simulink model "TosAeb"  (R2021a)
settings: Solver=FixedStepDiscrete  StartTime=0.0  StopTime=inf  FixedStep=0.01  RelTol=1e-3
undefined symbols (not defined in the file or in its folder): ACCModSts, AccSts1, AEB_ObjectMovingOrientation, AEB_ObjectMovingState, CN_BTCActive, CN_CurrentGearR, CN_CurrentGearR_DHT, CN_PTCActive, CN_VDCActive, CN_VSC_EspOff, CN_VSC_FCW_AEB_Error, CN_VSC_FCW_AEB_NoDispay, CN_VSC_FCW_AEB_PerfmDgdtn, CN_VSC_FCW_AEB_TempUnAvlbl, CN_VSC_VehStandstill, CNaccelAvoidMax, CNaccelAvoidMin, CNbrakePedalPress, CNcomfortStrLeftAccelMax, CNcomfortStrLeftAccelMin, CNcomfortStrRightAccelMax, CNcomfortStrRightAccelMin, CNDrvDoorOpen, CNDrvModeEco, CNDrvModeNorm, CNDrvModeOther, CNDrvModeSport, CNDrvSBRreminder, CNNull, CNobjInvalidID, CNPassDoorOpen, CNPassSBRreminder, CNPEBSAccelNegInf, CNPEBSAccelNull, CNPEBSTimeInf, CNPEBSTimeNull, CNSTOPMAX, CNSTOPNULL, CNsubjWidth, CNSwtOFF, CNSwtON, CNTTCMAX, CNTTCNULL, CNVehConfigAEBCycNone, CNVehConfigAEBNone, CNVehConfigAEBPedNone, CNVehConfigFCWNone, CNVehConfigJANone, CollisionSeverity, FALSE, FCWSnvty, JACycObjCalcInfoDefaultParams, JAPedObjCalcInfoDefaultParams, JAVehObjCalcInfoDefaultParams, latCycObjCalcInfoDefaultParams, latPedObjCalcInfoDefaultParams, longObjCalcInfoDefaultParams, ModSts, movingStatus, ObjectType ... (+1112)
libraries: simulink x3031, AEBSFunctionLib x50, Ta_Library x33, AEBSStateMachineLib x23, Mylibrary x12, simulink_extras x7, ObjSelect_lib x3, dspstat3 x2
(part 24 of 102)

== system TosAeb/FcwAebJa_Step_sys/ALG_AEBSystem/ALG_AEBController/AEB_FuncCondCalcApp/LongFunctionCondCalc/vehAEBpartSuppAborCondCalc/vehAEBpartSuppCondCalc ==
  inputSignals : Inport
  Bus Selector : BusSelector  OutputSignals=longObjCalcInfo.LongObjRawInfo.ObjectMovingState,longObjCalcInfo.LongObjRawInfo.ObjectTypeCameraConfirmed,longObjCalcInfo.LongObjRawInfo.ObjectType,subjInfo.subjCurvTraj,subjInfo.subjLatAccel,subjInfo.subjVel,vehConfigInfo.VehicleConfigAEBCyc,vehConfigInfo.VehicleConfigAEBPed,vehStateCalcInfo.VSC_AEBFuncSwtSts.AEB_PedResp,longObjCalcInfo.LongObjRawInfo.ObjectMovingOrientation,longObjCalcInfo.LongObjRawInfo.ObjectID,longObjCalcInfo.LongObjRawInfo.ObjectExistProb,longObjCalcInfo.LongObjRawInfo.ObjectIsObstacleProb,longObjCalcInfo.LongObjRawInfo.dxVariance,longObjCalcInfo.longObjAbsInfo.dxFrontBumper,longObjCalcInfo.longObjAbsInfo.ObjFusQuality
  Bus Selector1 : BusSelector  OutputSignals=subjInfo.subjVel,vehCanInfo.SteerWheelAngle,vehCanInfo.SteerWheelSpd,vehCanInfo.AccrPedlPosnDiagc,vehConfigInfo.VehConfigIbooster,vehCanInfo.ESP_MasterCylindBrakePress,vehCanInfo.BrakePedalStatus_G,vehStateCalcInfo.VSCVehSuppAborCond.VSCvehAEBpartSuppCond,vehCanInfo.IB_BrkPedalAppld
  Bus Selector2 : BusSelector  OutputSignals=longObjCalcInfo.LongObjRawInfo.ObjectTypeCameraConfirmed,longObjCalcInfo.longObjAbsInfo.dxFrontBumper,longObjCalcInfo.LongCollisionAssess.longCollisionSeverity,longObjCalcInfo.overlapRatio,LCK_Active,longObjCalcInfo.probEscape,subjInfo.subjCurvTraj
  vehAEBpartObjRelActiveCondCalc : Subsystem
  vehAEBpartSituationRelActiveCondCalc : Subsystem
  vehAEBpartSuppCondFinalJudge : Subsystem
  vehAEBpartVehRelActiveCondCalc : Subsystem
  vehAEBpartSuppCondFulfilled : Outport
  wires:
  Bus Selector1/5 -> vehAEBpartVehRelActiveCondCalc/5(VehConfigIbooster)  "<VehConfigIbooster>"
  Bus Selector/16 -> vehAEBpartObjRelActiveCondCalc/16(ObjFusQuality)  "<ObjFusQuality>"
  Bus Selector2/5 -> vehAEBpartSituationRelActiveCondCalc/5(LCK_Active)  "<LCK_Active>"
  Bus Selector1/9 -> vehAEBpartVehRelActiveCondCalc/9(IB_BrkPedalAppld)  "<IB_BrkPedalAppld>"
  Bus Selector2/7 -> vehAEBpartSituationRelActiveCondCalc/7(subjCurvTraj)  "<subjCurvTraj>"
  Bus Selector2/6 -> vehAEBpartSituationRelActiveCondCalc/6(probEscape)  "<probEscape>"
  Bus Selector2/4 -> vehAEBpartSituationRelActiveCondCalc/4(overlapRatio)  "<overlapRatio>"
  Bus Selector2/3 -> vehAEBpartSituationRelActiveCondCalc/3(longCollisionSeverity)  "<longCollisionSeverity>"
  Bus Selector2/2 -> vehAEBpartSituationRelActiveCondCalc/2(dxFrontBumper)  "<dxFrontBumper>"
  Bus Selector2/1 -> vehAEBpartSituationRelActiveCondCalc/1(ObjectTypeCameraConfirmed)  "<ObjectTypeCameraConfirmed>"
  Bus Selector1/1 -> vehAEBpartVehRelActiveCondCalc/1(subjVel)  "<subjVel>"
  Bus Selector1/2 -> vehAEBpartVehRelActiveCondCalc/2(SteerWheelAngle)  "<SteerWheelAngle>"
  Bus Selector1/8 -> vehAEBpartVehRelActiveCondCalc/8(vehStateCalcAEBpartSuppFlg)  "<VSCvehAEBpartSuppCond>"
  Bus Selector1/3 -> vehAEBpartVehRelActiveCondCalc/3(SteerWheelSpd)  "<SteerWheelSpd>"
  Bus Selector1/4 -> vehAEBpartVehRelActiveCondCalc/4(AccrPedlPosnDiagc)  "<AccrPedlPosnDiagc>"
  Bus Selector1/6 -> vehAEBpartVehRelActiveCondCalc/6(ESPmasterCylindBrakePress)  "<ESP_MasterCylindBrakePress>"
  Bus Selector1/7 -> vehAEBpartVehRelActiveCondCalc/7(BrakePedalStatus_G)  "<BrakePedalStatus_G>"
  Bus Selector/15 -> vehAEBpartObjRelActiveCondCalc/15(dxFrontBumper)  "<dxFrontBumper>"
  Bus Selector/1 -> vehAEBpartObjRelActiveCondCalc/1(ObjectMovingState)  "<ObjectMovingState>"
  Bus Selector/14 -> vehAEBpartObjRelActiveCondCalc/14(dxVariance)  "<dxVariance>"
  Bus Selector/2 -> vehAEBpartObjRelActiveCondCalc/2(ObjectTypeCameraConfirmed)  "<ObjectTypeCameraConfirmed>"
  Bus Selector/13 -> vehAEBpartObjRelActiveCondCalc/13(ObjectIsObstacleProb)  "<ObjectIsObstacleProb>"
  Bus Selector/3 -> vehAEBpartObjRelActiveCondCalc/3(ObjectType)  "<ObjectType>"
  Bus Selector/12 -> vehAEBpartObjRelActiveCondCalc/12(ObjectExistProb)  "<ObjectExistProb>"
  Bus Selector/4 -> vehAEBpartObjRelActiveCondCalc/4(subjCurvTraj)  "<subjCurvTraj>"
  Bus Selector/11 -> vehAEBpartObjRelActiveCondCalc/11(ObjectID)  "<ObjectID>"
  Bus Selector/5 -> vehAEBpartObjRelActiveCondCalc/5(subjLatAccel)  "<subjLatAccel>"
  Bus Selector/10 -> vehAEBpartObjRelActiveCondCalc/10(ObjectMovingOrientation)  "<ObjectMovingOrientation>"
  Bus Selector/6 -> vehAEBpartObjRelActiveCondCalc/6(subjVel)  "<subjVel>"
  Bus Selector/9 -> vehAEBpartObjRelActiveCondCalc/9(AEB_PedResp)  "<AEB_PedResp>"
  Bus Selector/7 -> vehAEBpartObjRelActiveCondCalc/7(vehConfigAEBCyc)  "<VehicleConfigAEBCyc>"
  Bus Selector/8 -> vehAEBpartObjRelActiveCondCalc/8(vehConfigAEBPed)  "<VehicleConfigAEBPed>"
  inputSignals/1 -> Bus Selector/1, Bus Selector1/1, Bus Selector2/1
  vehAEBpartSituationRelActiveCondCalc/1(vehAEBpartSituationRelActiveCondFulfilled) -> vehAEBpartSuppCondFinalJudge/3(vehAEBpartSituationRelActiveCondFulfilled)  "AEB_vehAEBpartSitRelActiveCond"
  vehAEBpartObjRelActiveCondCalc/1(vehAEBpartObjRelActiveCondFulfilled) -> vehAEBpartSuppCondFinalJudge/1(vehAEBpartObjRelActiveCondFulfilled)  "AEB_vehAEBpartObjRelActiveCond"
  vehAEBpartVehRelActiveCondCalc/1(vehAEBpartVehRelActiveCondFulfilled) -> vehAEBpartSuppCondFinalJudge/2(vehAEBpartVehRelActiveCondFulfilled)  "AEB_vehAEBpartVehRelActiveCond"
  vehAEBpartSuppCondFinalJudge/1(vehAEBpartSuppCondFulfilled) -> vehAEBpartSuppCondFulfilled/1

== system TosAeb/FcwAebJa_Step_sys/ALG_AEBSystem/ALG_AEBController/AEB_FuncCondCalcApp/LongFunctionCondCalc/vehAcuteWarningSuppAborCondFulfilled/vehAcuteWarningAborCondCalc ==
  inputSignals : Inport
  Bus Selector : BusSelector  OutputSignals=longObjCalcInfo.LongObjRawInfo.ObjectID
  Bus Selector1 : BusSelector  OutputSignals=vehCanInfo.SteerWheelAngle,vehCanInfo.SteerWheelSpd,vehCanInfo.ESP_MasterCylindBrakePress,vehStateCalcInfo.VSCVehSuppAborCond.VSCvehAcuteWarningAborCond
  Bus Selector2 : BusSelector  OutputSignals=longObjCalcInfo.overlapRatio,longObjCalcInfo.longObjAbsInfo.dxFrontBumper,longObjCalcInfo.probEscape
  vehAcuteWarningAborCondFinalJudge : Subsystem
  vehAcuteWarningObjRelAborCondCalc : Subsystem
  vehAcuteWarningSituationRelAborCondCalc : Subsystem
  vehAcuteWarningVehRelAborCondCalc : Subsystem
  vehAcuteWarningAborCond : Outport
  wires:
  Bus Selector2/1 -> vehAcuteWarningSituationRelAborCondCalc/1(overlapRatio)  "<overlapRatio>"
  Bus Selector2/3 -> vehAcuteWarningSituationRelAborCondCalc/3(probEscape)  "<probEscape>"
  Bus Selector2/2 -> vehAcuteWarningSituationRelAborCondCalc/2(dxFrontBumper)  "<dxFrontBumper>"
  Bus Selector1/4 -> vehAcuteWarningVehRelAborCondCalc/4(vehStateCalcAcuteWarningAborFlg)  "<VSCvehAcuteWarningAborCond>"
  Bus Selector1/3 -> vehAcuteWarningVehRelAborCondCalc/3(ESPmasterCylindBrakePress)  "<ESP_MasterCylindBrakePress>"
  Bus Selector1/1 -> vehAcuteWarningVehRelAborCondCalc/1(SteerWheelAngle)  "<SteerWheelAngle>"
  Bus Selector1/2 -> vehAcuteWarningVehRelAborCondCalc/2(SteerWheelSpd)  "<SteerWheelSpd>"
  Bus Selector/1 -> vehAcuteWarningObjRelAborCondCalc/1(ObjectID)  "<ObjectID>"
  inputSignals/1 -> Bus Selector1/1, Bus Selector2/1, Bus Selector/1
  vehAcuteWarningObjRelAborCondCalc/1(vehAcuteWarningObjRelAborCondFulfilled) -> vehAcuteWarningAborCondFinalJudge/1(vehAcuteWarningObjRelAborCondFulfilled)  "AEB_vehAcuteWarningObjRelAborCond"
  vehAcuteWarningVehRelAborCondCalc/1(vehAcuteWarningVehRelAborCondFulfilled) -> vehAcuteWarningAborCondFinalJudge/2(vehAcuteWarningVehRelAborCondFulfilled)  "AEB_vehAcuteWarningVehRelAborCond"
  vehAcuteWarningSituationRelAborCondCalc/1(vehAcuteWarningSituationRelAborCondFulfilled) -> vehAcuteWarningAborCondFinalJudge/3(vehAcuteWarningSituationRelAborCondFulfilled)  "AEB_vehAcuteWarningSituationRelAborCond"
  vehAcuteWarningAborCondFinalJudge/1(vehAcuteWarningAborCond) -> vehAcuteWarningAborCond/1

== system TosAeb/FcwAebJa_Step_sys/ALG_AEBSystem/ALG_AEBController/AEB_FuncCondCalcApp/LongFunctionCondCalc/vehAcuteWarningSuppAborCondFulfilled/vehAcuteWarningSuppCondCalc ==
  inputSignals : Inport
  Bus Selector : BusSelector  OutputSignals=longObjCalcInfo.LongObjRawInfo.ObjectMovingState,longObjCalcInfo.LongObjRawInfo.dz,longObjCalcInfo.LongObjRawInfo.ObjectTypeCameraConfirmed,longObjCalcInfo.LongObjRawInfo.ObjectType,subjInfo.subjCurvTraj,subjInfo.subjLatAccel,subjInfo.subjVel,vehConfigInfo.VehicleConfigAEBCyc,vehConfigInfo.VehicleConfigAEBPed,vehStateCalcInfo.VSC_AEBFuncSwtSts.AEB_PedResp,longObjCalcInfo.LongObjRawInfo.ObjectMovingOrientation,longObjCalcInfo.LongObjRawInfo.ObjectID,longObjCalcInfo.LongObjRawInfo.ObjectExistProb,longObjCalcInfo.LongObjRawInfo.ObjectIsObstacleProb,longObjCalcInfo.LongObjRawInfo.dxVariance,longObjCalcInfo.longObjAbsInfo.dxFrontBumper,longObjCalcInfo.longObjAbsInfo.ObjFusQuality
  Bus Selector1 : BusSelector  OutputSignals=subjInfo.subjVel,longObjCalcInfo.LongObjRawInfo.ObjectMovingState,vehCanInfo.SteerWheelAngle,vehCanInfo.SteerWheelSpd,vehCanInfo.AccrPedlPosnDiagc,vehConfigInfo.VehConfigIbooster,vehCanInfo.ESP_MasterCylindBrakePress,vehCanInfo.BrakePedalStatus_G,vehCanInfo.IB_BrkPedalAppld,vehStateCalcInfo.VSCVehSuppAborCond.VSCvehPreWarningSuppCond,subjInfo.subjLongAccel
  Bus Selector2 : BusSelector  OutputSignals=longObjCalcInfo.LongCollisionAssess.longCollisionSeverity,longObjCalcInfo.overlapRatio,longObjCalcInfo.longObjAbsInfo.dxFrontBumper,longObjCalcInfo.probEscape,subjInfo.subjCurvTraj
  vehAcuteWarningObjRelActiveCondCalc : Subsystem
  vehAcuteWarningSituationRelActiveCondCalc : Subsystem
  vehAcuteWarningSuppCondFinalJudge : Subsystem
  vehAcuteWarningVehRelActiveCondCalc : Subsystem
  vehAcuteWarningSuppCond : Outport
  wires:
  Bus Selector1/6 -> vehAcuteWarningVehRelActiveCondCalc/6(VehConfigIbooster)  "<VehConfigIbooster>"
  Bus Selector/17 -> vehAcuteWarningObjRelActiveCondCalc/17(ObjFusQuality)  "<ObjFusQuality>"
  Bus Selector1/9 -> vehAcuteWarningVehRelActiveCondCalc/9(IB_BrkPedalAppld)  "<IB_BrkPedalAppld>"
  Bus Selector2/5 -> vehAcuteWarningSituationRelActiveCondCalc/5(subjCurvTraj)  "<subjCurvTraj>"
  Bus Selector2/1 -> vehAcuteWarningSituationRelActiveCondCalc/1(longCollisionSeverity)  "<longCollisionSeverity>"
  Bus Selector2/4 -> vehAcuteWarningSituationRelActiveCondCalc/4(probEscape)  "<probEscape>"
  Bus Selector2/2 -> vehAcuteWarningSituationRelActiveCondCalc/2(overlapRatio)  "<overlapRatio>"
  Bus Selector2/3 -> vehAcuteWarningSituationRelActiveCondCalc/3(dxFrontBumper)  "<dxFrontBumper>"
  Bus Selector1/1 -> vehAcuteWarningVehRelActiveCondCalc/1(subjVel)  "<subjVel>"
  Bus Selector1/2 -> vehAcuteWarningVehRelActiveCondCalc/2(ObjectMovingState)  "<ObjectMovingState>"
  Bus Selector1/11 -> vehAcuteWarningVehRelActiveCondCalc/11(subjLongAccel)  "<subjLongAccel>"
  Bus Selector1/3 -> vehAcuteWarningVehRelActiveCondCalc/3(SteerWheelAngle)  "<SteerWheelAngle>"
  Bus Selector1/10 -> vehAcuteWarningVehRelActiveCondCalc/10(vehStateCalcPreWarningSuppFlg)  "<VSCvehPreWarningSuppCond>"
  Bus Selector1/4 -> vehAcuteWarningVehRelActiveCondCalc/4(SteerWheelSpd)  "<SteerWheelSpd>"
  Bus Selector1/5 -> vehAcuteWarningVehRelActiveCondCalc/5(AccrPedlPosnDiagc)  "<AccrPedlPosnDiagc>"
  Bus Selector1/7 -> vehAcuteWarningVehRelActiveCondCalc/7(ESPmasterCylindBrakePress)  "<ESP_MasterCylindBrakePress>"
  Bus Selector1/8 -> vehAcuteWarningVehRelActiveCondCalc/8(BrakePedalStatus_G)  "<BrakePedalStatus_G>"
  Bus Selector/16 -> vehAcuteWarningObjRelActiveCondCalc/16(dxFrontBumper)  "<dxFrontBumper>"
  Bus Selector/15 -> vehAcuteWarningObjRelActiveCondCalc/15(dxVariance)  "<dxVariance>"
  Bus Selector/14 -> vehAcuteWarningObjRelActiveCondCalc/14(ObjectIsObstacleProb)  "<ObjectIsObstacleProb>"
  Bus Selector/13 -> vehAcuteWarningObjRelActiveCondCalc/13(ObjectExistProb)  "<ObjectExistProb>"
  Bus Selector/12 -> vehAcuteWarningObjRelActiveCondCalc/12(ObjectID)  "<ObjectID>"
  Bus Selector/11 -> vehAcuteWarningObjRelActiveCondCalc/11(ObjectMovingOrientation)  "<ObjectMovingOrientation>"
  Bus Selector/10 -> vehAcuteWarningObjRelActiveCondCalc/10(AEB_PedResp)  "<AEB_PedResp>"
  Bus Selector/9 -> vehAcuteWarningObjRelActiveCondCalc/9(vehConfigAEBPed)  "<VehicleConfigAEBPed>"
  Bus Selector/8 -> vehAcuteWarningObjRelActiveCondCalc/8(vehConfigAEBCyc)  "<VehicleConfigAEBCyc>"
  Bus Selector/7 -> vehAcuteWarningObjRelActiveCondCalc/7(subjVel)  "<subjVel>"
  Bus Selector/1 -> vehAcuteWarningObjRelActiveCondCalc/1(ObjectMovingState)  "<ObjectMovingState>"
  Bus Selector/6 -> vehAcuteWarningObjRelActiveCondCalc/6(subjLatAccel)  "<subjLatAccel>"
  Bus Selector/2 -> vehAcuteWarningObjRelActiveCondCalc/2(dz)  "<dz>"
  Bus Selector/5 -> vehAcuteWarningObjRelActiveCondCalc/5(subjCurvTraj)  "<subjCurvTraj>"
  Bus Selector/3 -> vehAcuteWarningObjRelActiveCondCalc/3(ObjectTypeCameraConfirmed)  "<ObjectTypeCameraConfirmed>"
  Bus Selector/4 -> vehAcuteWarningObjRelActiveCondCalc/4(ObjectType)  "<ObjectType>"
  inputSignals/1 -> Bus Selector/1, Bus Selector1/1, Bus Selector2/1
  vehAcuteWarningSuppCondFinalJudge/1(vehAcuteWarningSuppCond) -> vehAcuteWarningSuppCond/1  "vehAcuteWarningSuppCond"
  vehAcuteWarningVehRelActiveCondCalc/1(vehAcuteWarningVehRelActiveCondFulfilled) -> vehAcuteWarningSuppCondFinalJudge/2(vehAcuteWarningVehRelActiveCondFulfilled)  "AEB_vehAcuteWarningVehRelActiveCond"
  vehAcuteWarningObjRelActiveCondCalc/1(vehAcuteWarningObjRelActiveCondFulfilled) -> vehAcuteWarningSuppCondFinalJudge/1(vehAcuteWarningObjRelActiveCondFulfilled)  "AEB_vehAcuteWarningObjRelActiveCond"
  vehAcuteWarningSituationRelActiveCondCalc/1(vehAcuteWarningSituationRelActiveCondFulfilled) -> vehAcuteWarningSuppCondFinalJudge/3(vehAcuteWarningSituationRelActiveCondFulfilled)  "AEB_vehAcuteWarningSituationRelActiveCond"

== system TosAeb/FcwAebJa_Step_sys/ALG_AEBSystem/ALG_AEBController/AEB_FuncCondCalcApp/LongFunctionCondCalc/vehEBASuppAborCondCalc/vehEBAAborCondCalc ==
  inputSignals : Inport
  Bus Selector : BusSelector  OutputSignals=longObjCalcInfo.LongObjRawInfo.ObjectID
  Bus Selector1 : BusSelector  OutputSignals=vehCanInfo.BrakePedalStatus_G,vehCanInfo.ESP_MasterCylindBrakePress,vehCanInfo.SteerWheelAngle,vehCanInfo.SteerWheelSpd,vehCanInfo.AccrPedlPosnDiagc,vehStateCalcInfo.VSCVehSuppAborCond.VSCvehEBAAborCond
  vehEBAAborCondFinalJudge : Subsystem
  vehEBAObjRelAborCondCalc : Subsystem
  vehEBASituationRelAborCondCalc : Subsystem
  vehEBAVehRelAborCondCalc : Subsystem
  vehEBAAborCondNoStandstillFulfilled : Outport
  vehEBAAborCondStandstillFulfilled : Outport  Port=2
  wires:
  Bus Selector1/6 -> vehEBAVehRelAborCondCalc/6(vehStateCalcEBAAborFlg)  "<VSCvehEBAAborCond>"
  Bus Selector1/1 -> vehEBAVehRelAborCondCalc/1(BrakePedalStatus_G)  "<BrakePedalStatus_G>"
  Bus Selector1/5 -> vehEBAVehRelAborCondCalc/5(AccrPedlPosnDiagc)  "<AccrPedlPosnDiagc>"
  Bus Selector1/2 -> vehEBAVehRelAborCondCalc/2(ESPmasterCylindBrakePress)  "<ESP_MasterCylindBrakePress>"
  Bus Selector1/4 -> vehEBAVehRelAborCondCalc/4(SteerWheelSpd)  "<SteerWheelSpd>"
  Bus Selector1/3 -> vehEBAVehRelAborCondCalc/3(SteerWheelAngle)  "<SteerWheelAngle>"
  Bus Selector/1 -> vehEBAObjRelAborCondCalc/1(ObjectID)  "<ObjectID>"
  inputSignals/1 -> Bus Selector1/1, Bus Selector/1
  vehEBAAborCondFinalJudge/2(vehEBAAborCondStandstillFulfilled) -> vehEBAAborCondStandstillFulfilled/1
  vehEBAAborCondFinalJudge/1(vehEBAAborCondNoStandstillFulfilled) -> vehEBAAborCondNoStandstillFulfilled/1
  vehEBASituationRelAborCondCalc/1(vehEBASituationRelAborCondFulfilled) -> vehEBAAborCondFinalJudge/3(vehEBASituationRelAborCondFulfilled)  "AEB_vehEBASituationRelAborCond"
  vehEBAVehRelAborCondCalc/1(vehEBAVehRelAborCondFulfilled) -> vehEBAAborCondFinalJudge/2(vehEBAVehRelAborCondFulfilled)  "AEB_vehEBAVehRelAborCond"
  vehEBAObjRelAborCondCalc/1(vehEBAObjRelAborCondFulfilled) -> vehEBAAborCondFinalJudge/1(vehEBAObjRelAborCondFulfilled)  "AEB_vehEBAObjRelAborCond"

== system TosAeb/FcwAebJa_Step_sys/ALG_AEBSystem/ALG_AEBController/AEB_FuncCondCalcApp/LongFunctionCondCalc/vehEBASuppAborCondCalc/vehEBASuppCondCalc ==
  inputSignals : Inport
  Bus Selector : BusSelector  OutputSignals=longObjCalcInfo.LongObjRawInfo.ObjectMovingState,longObjCalcInfo.longObjAbsInfo.dxFrontBumper,longObjCalcInfo.LongObjRawInfo.ObjectTypeCameraConfirmed,longObjCalcInfo.LongObjRawInfo.ObjectType,subjInfo.subjCurvTraj,subjInfo.subjLatAccel,subjInfo.subjVel,vehConfigInfo.VehicleConfigAEBCyc,vehConfigInfo.VehicleConfigAEBPed,vehStateCalcInfo.VSC_AEBFuncSwtSts.AEB_PedResp,longObjCalcInfo.LongObjRawInfo.ObjectMovingOrientation,longObjCalcInfo.LongObjRawInfo.ObjectID,longObjCalcInfo.LongObjRawInfo.ObjectExistProb,longObjCalcInfo.LongObjRawInfo.ObjectIsObstacleProb,longObjCalcInfo.LongObjRawInfo.dxVariance,longObjCalcInfo.longObjAbsInfo.ObjFusQuality
  Bus Selector1 : BusSelector  OutputSignals=subjInfo.subjVel,longObjCalcInfo.LongObjRawInfo.ObjectMovingState,vehStateCalcInfo.VSCVehSuppAborCond.VSCvehEBASuppCond,vehCanInfo.SteerWheelAngle,vehCanInfo.SteerWheelSpd,vehCanInfo.AccrPedlPosnDiagc
  Bus Selector2 : BusSelector  OutputSignals=longObjCalcInfo.LongObjRawInfo.ObjectTypeCameraConfirmed,longObjCalcInfo.longObjAbsInfo.dxFrontBumper,vehConfigInfo.VehConfigIbooster,longObjCalcInfo.LongCollisionAssess.accelAvoidHighLvlBrk4Brake,longObjCalcInfo.LongObjRawInfo.ObjectMovingState,subjInfo.subjVel,vehCanInfo.ESP_MasterCylindBrakePress,vehCanInfo.BrakePedalStatus_G,vehCanInfo.LongitudeAcc,subjInfo.subjLongAccel,AEBModStsFuncImpArb.warningFuncImpArb.preWarningFuncImpArb,longObjCalcInfo.overlapRatio,longObjCalcInfo.LongCollisionAssess.longCollisionSeverity,vehCanInfo.IB_BrkPedalAppld
  vehEBAObjRelActiveCondCalc : Subsystem
  vehEBASituationRelActiveCondCalc : Subsystem
  vehEBASuppCondFinalJudge : Subsystem
  vehEBAVehRelActiveCondCalc : Subsystem
  vehEBASuppCondFulfilled : Outport
  wires:
  Bus Selector2/3 -> vehEBASituationRelActiveCondCalc/3(VehConfigIbooster)  "<VehConfigIbooster>"
  Bus Selector/16 -> vehEBAObjRelActiveCondCalc/16(ObjFusQuality)  "<ObjFusQuality>"
  Bus Selector2/14 -> vehEBASituationRelActiveCondCalc/14(IB_BrkPedalAppld)  "<IB_BrkPedalAppld>"
  Bus Selector2/13 -> vehEBASituationRelActiveCondCalc/13(longCollisionSeverity)  "<longCollisionSeverity>"
  Bus Selector2/11 -> vehEBASituationRelActiveCondCalc/11(PreWarningFuncImpArb)  "<preWarningFuncImpArb>"
  Bus Selector2/12 -> vehEBASituationRelActiveCondCalc/12(overlapRatio)  "<overlapRatio>"
  Bus Selector2/10 -> vehEBASituationRelActiveCondCalc/10(subjLongAccel)  "<subjLongAccel>"
  Bus Selector2/9 -> vehEBASituationRelActiveCondCalc/9(subjLongAccelFromSensor)  "<LongitudeAcc>"
  Bus Selector2/8 -> vehEBASituationRelActiveCondCalc/8(BrakePedalStatus_G)  "<BrakePedalStatus_G>"
  Bus Selector2/1 -> vehEBASituationRelActiveCondCalc/1(ObjectTypeCameraConfirmed)  "<ObjectTypeCameraConfirmed>"
  Bus Selector2/7 -> vehEBASituationRelActiveCondCalc/7(ESPmasterCylindBrakePress)  "<ESP_MasterCylindBrakePress>"
  Bus Selector2/2 -> vehEBASituationRelActiveCondCalc/2(dxFrontBumper)  "<dxFrontBumper>"
  Bus Selector2/6 -> vehEBASituationRelActiveCondCalc/6(subjVel)  "<subjVel>"
  Bus Selector2/4 -> vehEBASituationRelActiveCondCalc/4(predictiveAccelAvoid)  "<accelAvoidHighLvlBrk4Brake>"
  Bus Selector2/5 -> vehEBASituationRelActiveCondCalc/5(ObjectMovingState)  "<ObjectMovingState>"
  Bus Selector1/6 -> vehEBAVehRelActiveCondCalc/6(AccrPedlPosnDiagc)  "<AccrPedlPosnDiagc>"
  Bus Selector1/5 -> vehEBAVehRelActiveCondCalc/5(SteerWheelSpd)  "<SteerWheelSpd>"
  Bus Selector1/1 -> vehEBAVehRelActiveCondCalc/1(subjVel)  "<subjVel>"
  Bus Selector1/4 -> vehEBAVehRelActiveCondCalc/4(SteerWheelAngle)  "<SteerWheelAngle>"
  Bus Selector1/2 -> vehEBAVehRelActiveCondCalc/2(ObjectMovingState)  "<ObjectMovingState>"
  Bus Selector1/3 -> vehEBAVehRelActiveCondCalc/3(vehStateCalcEBASuppFlg)  "<VSCvehEBASuppCond>"
  Bus Selector/15 -> vehEBAObjRelActiveCondCalc/15(dxVariance)  "<dxVariance>"
  Bus Selector/1 -> vehEBAObjRelActiveCondCalc/1(ObjectMovingState)  "<ObjectMovingState>"
  Bus Selector/14 -> vehEBAObjRelActiveCondCalc/14(ObjectIsObstacleProb)  "<ObjectIsObstacleProb>"
  Bus Selector/2 -> vehEBAObjRelActiveCondCalc/2(dxFrontBumper)  "<dxFrontBumper>"
  Bus Selector/13 -> vehEBAObjRelActiveCondCalc/13(ObjectExistProb)  "<ObjectExistProb>"
  Bus Selector/3 -> vehEBAObjRelActiveCondCalc/3(ObjectTypeCameraConfirmed)  "<ObjectTypeCameraConfirmed>"
  Bus Selector/12 -> vehEBAObjRelActiveCondCalc/12(ObjectID)  "<ObjectID>"
  Bus Selector/4 -> vehEBAObjRelActiveCondCalc/4(ObjectType)  "<ObjectType>"
  Bus Selector/11 -> vehEBAObjRelActiveCondCalc/11(ObjectMovingOrientation)  "<ObjectMovingOrientation>"
  Bus Selector/5 -> vehEBAObjRelActiveCondCalc/5(subjCurvTraj)  "<subjCurvTraj>"
  Bus Selector/10 -> vehEBAObjRelActiveCondCalc/10(AEB_PedResp)  "<AEB_PedResp>"
  Bus Selector/6 -> vehEBAObjRelActiveCondCalc/6(subjLatAccel)  "<subjLatAccel>"
  Bus Selector/9 -> vehEBAObjRelActiveCondCalc/9(vehConfigAEBPed)  "<VehicleConfigAEBPed>"
  Bus Selector/7 -> vehEBAObjRelActiveCondCalc/7(subjVel)  "<subjVel>"
  Bus Selector/8 -> vehEBAObjRelActiveCondCalc/8(vehConfigAEBCyc)  "<VehicleConfigAEBCyc>"
  inputSignals/1 -> Bus Selector/1, Bus Selector1/1, Bus Selector2/1
  vehEBASituationRelActiveCondCalc/1(vehEBASituationRelActiveCondFulfilled) -> vehEBASuppCondFinalJudge/3(vehEBASituationRelActiveCondFulfilled)  "AEB_vehEBASitRelActiveCond"
  vehEBAObjRelActiveCondCalc/1(vehEBAObjRelActiveCondFulfilled) -> vehEBASuppCondFinalJudge/1(vehEBAObjRelActiveCondFulfilled)  "AEB_vehEBAObjRelActiveCond"
  vehEBAVehRelActiveCondCalc/1(vehEBAVehRelActiveCondFulfilled) -> vehEBASuppCondFinalJudge/2(vehEBAVehRelActiveCondFulfilled)  "AEB_vehEBAVehRelActiveCond"
  vehEBASuppCondFinalJudge/1(vehEBASuppCondFulfilled) -> vehEBASuppCondFulfilled/1  "vehAEBfullSuppCondFulfilled"

== system TosAeb/FcwAebJa_Step_sys/ALG_AEBSystem/ALG_AEBController/AEB_FuncCondCalcApp/LongFunctionCondCalc/vehLatentWarningSuppAborCondFulfilled/vehLatentWarningAborCondCalc ==
  inputSignals : Inport
  Bus Selector : BusSelector  OutputSignals=longObjCalcInfo.LongObjRawInfo.ObjectID
  Bus Selector1 : BusSelector  OutputSignals=vehStateCalcInfo.VSCVehSuppAborCond.VSCvehLatentWarningAborCond,subjInfo.subjVel,ACCModSts
  Bus Selector2 : BusSelector  OutputSignals=subjInfo.subjVel,longObjCalcInfo.longObjAbsInfo.dxFrontBumper
  vehLatentWarningAborCondFinalJudge : Subsystem
  vehLatentWarningObjRelActiveCondCalc : Subsystem
  vehLatentWarningSituationRelAborCondCalc : Subsystem
  vehLatentWarningVehRelActiveCondCalc : Subsystem
  vehLatentWarningAborCondFulfilled : Outport
  wires:
  Bus Selector1/2 -> vehLatentWarningVehRelActiveCondCalc/2(subjVel)  "<subjVel>"
  Bus Selector1/1 -> vehLatentWarningVehRelActiveCondCalc/1(vehStateCalcLatentWarningAborFlg)  "<VSCvehLatentWarningAborCond>"
  Bus Selector1/3 -> vehLatentWarningVehRelActiveCondCalc/3(ACCModSts)  "<ACCModSts>"
  Bus Selector2/2 -> vehLatentWarningSituationRelAborCondCalc/2(dxFrontBumper)  "<dxFrontBumper>"
  Bus Selector2/1 -> vehLatentWarningSituationRelAborCondCalc/1(subjVel)  "<subjVel>"
  Bus Selector/1 -> vehLatentWarningObjRelActiveCondCalc/1(ObjectID)  "<ObjectID>"
  inputSignals/1 -> Bus Selector/1, Bus Selector1/1, Bus Selector2/1
  vehLatentWarningObjRelActiveCondCalc/1(vehLatentWarningObjRelActiveCondFulfilled) -> vehLatentWarningAborCondFinalJudge/1(vehLatentWarningObjRelAborCondFulfilled)  "AEB_vehLatentWarningObjRelAborCond"
  vehLatentWarningVehRelActiveCondCalc/1(vehLatentWarningVehRelAborCondFulfilled) -> vehLatentWarningAborCondFinalJudge/2(vehLatentWarningVehRelAborCondFulfilled)  "AEB_vehLatentWarningVehRelAborCond"
  vehLatentWarningSituationRelAborCondCalc/1(vehLatentWarningSituationRelAborCondFulfilled) -> vehLatentWarningAborCondFinalJudge/3(vehLatentWarningSituationRelAborCondFulfilled)  "AEB_vehLatentWarningSitRelAborCond"
  vehLatentWarningAborCondFinalJudge/1(vehLatentWarningAborCondFulfilled) -> vehLatentWarningAborCondFulfilled/1  "vehPreWarningAborCondFulfilled"

== system TosAeb/FcwAebJa_Step_sys/ALG_AEBSystem/ALG_AEBController/AEB_FuncCondCalcApp/LongFunctionCondCalc/vehLatentWarningSuppAborCondFulfilled/vehLatentWarningSuppCondCalc ==
  inputSignals : Inport
  Bus Selector : BusSelector  OutputSignals=longObjCalcInfo.LongObjRawInfo.ObjectMovingState,longObjCalcInfo.LongObjRawInfo.ObjectType,longObjCalcInfo.LongObjRawInfo.ObjectMovingOrientation,longObjCalcInfo.LongObjRawInfo.ObjectID,longObjCalcInfo.LongObjRawInfo.ObjectExistProb,longObjCalcInfo.LongObjRawInfo.ObjectIsObstacleProb
  Bus Selector1 : BusSelector  OutputSignals=subjInfo.subjVel,ACCModSts,vehStateCalcInfo.VSCVehSuppAborCond.VSCvehLatentWarningSuppCond
  Bus Selector2 : BusSelector  OutputSignals=subjInfo.subjVel,longObjCalcInfo.longObjAbsInfo.dxFrontBumper,vehStateCalcInfo.VSC_AEBFuncSwtSts.FCWSnvty
  vehLatentWarningObjRelActiveCondCalc : Subsystem
  vehLatentWarningSituationRelActiveCondCalc : Subsystem
  vehLatentWarningSuppCondFinalJudge : Subsystem
  vehLatentWarningVehRelActiveCondCalc : Subsystem
  vehLatentWarningSuppCondFulfilled : Outport
  wires:
  Bus Selector2/1 -> vehLatentWarningSituationRelActiveCondCalc/1(subjVel)  "<subjVel>"
  Bus Selector2/3 -> vehLatentWarningSituationRelActiveCondCalc/3(FCWSnvty)  "<FCWSnvty>"
  Bus Selector2/2 -> vehLatentWarningSituationRelActiveCondCalc/2(dxFrontBumper)  "<dxFrontBumper>"
  Bus Selector1/3 -> vehLatentWarningVehRelActiveCondCalc/3(vehStateCalcLatentWarningSuppFlg)  "<VSCvehLatentWarningSuppCond>"
  Bus Selector1/2 -> vehLatentWarningVehRelActiveCondCalc/2(ACCModSts)  "<ACCModSts>"
  Bus Selector1/1 -> vehLatentWarningVehRelActiveCondCalc/1(subjVel)  "<subjVel>"
  Bus Selector/6 -> vehLatentWarningObjRelActiveCondCalc/6(ObjectIsObstacleProb)  "<ObjectIsObstacleProb>"
  Bus Selector/5 -> vehLatentWarningObjRelActiveCondCalc/5(ObjectExistProb)  "<ObjectExistProb>"
  Bus Selector/4 -> vehLatentWarningObjRelActiveCondCalc/4(ObjectID)  "<ObjectID>"
  Bus Selector/1 -> vehLatentWarningObjRelActiveCondCalc/1(ObjectMovingState)  "<ObjectMovingState>"
  Bus Selector/3 -> vehLatentWarningObjRelActiveCondCalc/3(ObjectMovingOrientation)  "<ObjectMovingOrientation>"
  Bus Selector/2 -> vehLatentWarningObjRelActiveCondCalc/2(ObjectType)  "<ObjectType>"
  inputSignals/1 -> Bus Selector/1, Bus Selector1/1, Bus Selector2/1
  vehLatentWarningSuppCondFinalJudge/1(vehLatentWarningAborCondFulfilled) -> vehLatentWarningSuppCondFulfilled/1
  vehLatentWarningVehRelActiveCondCalc/1(vehLatentWarningVehRelActiveCondFulfilled) -> vehLatentWarningSuppCondFinalJudge/2(vehLatentWarningVehRelActiveCondFulfilled)  "AEB_vehLatentWarningVehRelActiveCond"
  vehLatentWarningObjRelActiveCondCalc/1(vehLatentWarningObjRelActiveCondFulfilled) -> vehLatentWarningSuppCondFinalJudge/1(vehLatentWarningObjRelActiveCondFulfilled)  "AEB_vehLatentWarningObjRelActiveCond"
  vehLatentWarningSituationRelActiveCondCalc/1(vehLatentWarningSituationRelActiveCondFulfilled) -> vehLatentWarningSuppCondFinalJudge/3(vehLatentWarningSituationRelActiveCondFulfilled)  "AEB_vehLatentWarningSitRelActiveCond"

== system TosAeb/FcwAebJa_Step_sys/ALG_AEBSystem/ALG_AEBController/AEB_FuncCondCalcApp/LongFunctionCondCalc/vehPreWarningSuppAborCondFulfilled/vehPreWarningAborCondCalc ==
  inputSignals : Inport
  Bus Selector : BusSelector  OutputSignals=longObjCalcInfo.LongObjRawInfo.ObjectID
  Bus Selector1 : BusSelector  OutputSignals=vehCanInfo.SteerWheelAngle,vehCanInfo.SteerWheelSpd,vehCanInfo.ESP_MasterCylindBrakePress,vehStateCalcInfo.VSCVehSuppAborCond.VSCvehPreWarningAborCond,AEBModStsFuncImpArb.brkFuncImpArb.EBAFuncImpArb
  Bus Selector2 : BusSelector  OutputSignals=longObjCalcInfo.LongCollisionAssess.longCollisionSeverity
  vehPreWarningAborCondFinalJudge : Subsystem
  vehPreWarningObjRelAborCondCalc : Subsystem
  vehPreWarningSituationRelAborCondCalc : Subsystem
  vehPreWarningVehRelActiveCondCalc : Subsystem
  vehPreWarningAborCond : Outport
  wires:
  Bus Selector2/1 -> vehPreWarningSituationRelAborCondCalc/1(longCollisionSeverity)  "<longCollisionSeverity>"
  Bus Selector1/5 -> vehPreWarningVehRelActiveCondCalc/5(EBAFuncImpArb)  "<EBAFuncImpArb>"
  Bus Selector1/1 -> vehPreWarningVehRelActiveCondCalc/1(SteerWheelAngle)  "<SteerWheelAngle>"
  Bus Selector1/4 -> vehPreWarningVehRelActiveCondCalc/4(vehStateCalcPreWarningAborFlg)  "<VSCvehPreWarningAborCond>"
  Bus Selector1/2 -> vehPreWarningVehRelActiveCondCalc/2(SteerWheelSpd)  "<SteerWheelSpd>"
  Bus Selector1/3 -> vehPreWarningVehRelActiveCondCalc/3(ESPmasterCylindBrakePress)  "<ESP_MasterCylindBrakePress>"
  Bus Selector/1 -> vehPreWarningObjRelAborCondCalc/1(ObjectID)  "<ObjectID>"
  inputSignals/1 -> Bus Selector/1, Bus Selector1/1, Bus Selector2/1
  vehPreWarningAborCondFinalJudge/1(vehPreWarningAborCond) -> vehPreWarningAborCond/1  "vehPreWarningAborCond"
  vehPreWarningSituationRelAborCondCalc/1(vehPreWarningSituationRelAborCondFulfilled) -> vehPreWarningAborCondFinalJudge/3(vehPreWarningSituationRelAborCondFulfilled)  "AEB_vehPreWarningSitRelAborCond"
  vehPreWarningVehRelActiveCondCalc/1(vehPreWarningVehRelAborCondFulfilled) -> vehPreWarningAborCondFinalJudge/2(vehPreWarningVehRelAborCondFulfilled)  "AEB_vehPreWarningVehRelAborCond"
  vehPreWarningObjRelAborCondCalc/1(vehPreWarningObjRelAborCondFulfilled) -> vehPreWarningAborCondFinalJudge/1(vehPreWarningObjRelAborCondFulfilled)  "AEB_vehPreWarningObjRelAborCond"

== system TosAeb/FcwAebJa_Step_sys/ALG_AEBSystem/ALG_AEBController/AEB_FuncCondCalcApp/LongFunctionCondCalc/vehPreWarningSuppAborCondFulfilled/vehPreWarningSuppCondCalc ==
  inputSignals : Inport
  Bus Selector : BusSelector  OutputSignals=longObjCalcInfo.LongObjRawInfo.ObjectMovingState,longObjCalcInfo.LongObjRawInfo.dz,longObjCalcInfo.LongObjRawInfo.ObjectTypeCameraConfirmed,vehStateCalcInfo.VSC_AEBFuncSwtSts.AEB_PedResp,longObjCalcInfo.LongObjRawInfo.ObjectType,subjInfo.subjCurvTraj,subjInfo.subjLatAccel,subjInfo.subjVel,vehConfigInfo.VehicleConfigAEBCyc,vehConfigInfo.VehicleConfigAEBPed,longObjCalcInfo.LongObjRawInfo.ObjectMovingOrientation,longObjCalcInfo.LongObjRawInfo.ObjectID,longObjCalcInfo.LongObjRawInfo.ObjectExistProb,longObjCalcInfo.LongObjRawInfo.ObjectIsObstacleProb,longObjCalcInfo.LongObjRawInfo.dxVariance,longObjCalcInfo.longObjAbsInfo.dxFrontBumper,longObjCalcInfo.longObjAbsInfo.ObjFusQuality
  Bus Selector1 : BusSelector  OutputSignals=subjInfo.subjVel,longObjCalcInfo.LongObjRawInfo.ObjectMovingState,vehStateCalcInfo.VSCVehSuppAborCond.VSCvehPreWarningSuppCond,vehCanInfo.SteerWheelAngle,vehCanInfo.SteerWheelSpd,vehConfigInfo.VehConfigIbooster,vehCanInfo.ESP_MasterCylindBrakePress,vehCanInfo.BrakePedalStatus_G,vehCanInfo.IB_BrkPedalAppld,vehCanInfo.AccrPedlPosnDiagc
  Bus Selector2 : BusSelector  OutputSignals=longObjCalcInfo.LongCollisionAssess.longCollisionSeverity,longObjCalcInfo.overlapRatio,longObjCalcInfo.longObjAbsInfo.dxFrontBumper,longObjCalcInfo.probEscape,subjInfo.subjCurvTraj,longObjCalcInfo.LongCollisionAssess.TTC,vehStateCalcInfo.VSC_AEBFuncSwtSts.FCWSnvty,subjInfo.subjVel,ACCModSts
  vehPreWarningObjRelActiveCondCalc : Subsystem
  vehPreWarningSituationRelActiveCondCalc : Subsystem
  vehPreWarningSuppCondFinalJudge : Subsystem
  vehPreWarningVehRelActiveCondCalc : Subsystem
  vehPreWarningSuppCond : Outport
  wires:
  Bus Selector1/6 -> vehPreWarningVehRelActiveCondCalc/6(VehConfigIbooster)  "<VehConfigIbooster>"
  Bus Selector/17 -> vehPreWarningObjRelActiveCondCalc/17(ObjFusQuality)  "<ObjFusQuality>"
  Bus Selector2/8 -> vehPreWarningSituationRelActiveCondCalc/8(subjVel)  "<subjVel>"
  Bus Selector2/6 -> vehPreWarningSituationRelActiveCondCalc/6(TTC)  "<TTC>"
  Bus Selector1/9 -> vehPreWarningVehRelActiveCondCalc/9(IB_BrkPedalAppld)  "<IB_BrkPedalAppld>"
  Bus Selector2/3 -> vehPreWarningSituationRelActiveCondCalc/3(dxFrontBumper)  "<dxFrontBumper>"
  Bus Selector2/5 -> vehPreWarningSituationRelActiveCondCalc/5(subjCurvTraj)  "<subjCurvTraj>"
  Bus Selector2/2 -> vehPreWarningSituationRelActiveCondCalc/2(overlapRatio)  "<overlapRatio>"
  Bus Selector2/4 -> vehPreWarningSituationRelActiveCondCalc/4(probEscape)  "<probEscape>"
  Bus Selector2/1 -> vehPreWarningSituationRelActiveCondCalc/1(longCollisionSeverity)  "<longCollisionSeverity>"
  Bus Selector/5 -> vehPreWarningObjRelActiveCondCalc/5(ObjectType)  "<ObjectType>"
  Bus Selector/9 -> vehPreWarningObjRelActiveCondCalc/9(vehConfigAEBCyc)  "<VehicleConfigAEBCyc>"
  Bus Selector/2 -> vehPreWarningObjRelActiveCondCalc/2(dz)  "<dz>"
  Bus Selector/4 -> vehPreWarningObjRelActiveCondCalc/4(AEB_PedResp)  "<AEB_PedResp>"
  Bus Selector/14 -> vehPreWarningObjRelActiveCondCalc/14(ObjectIsObstacleProb)  "<ObjectIsObstacleProb>"
  Bus Selector/8 -> vehPreWarningObjRelActiveCondCalc/8(subjVel)  "<subjVel>"
  Bus Selector/16 -> vehPreWarningObjRelActiveCondCalc/16(dxFrontBumper)  "<dxFrontBumper>"
  Bus Selector/13 -> vehPreWarningObjRelActiveCondCalc/13(ObjectExistProb)  "<ObjectExistProb>"
  Bus Selector/7 -> vehPreWarningObjRelActiveCondCalc/7(subjLatAccel)  "<subjLatAccel>"
  Bus Selector/6 -> vehPreWarningObjRelActiveCondCalc/6(subjCurvTraj)  "<subjCurvTraj>"
  Bus Selector/1 -> vehPreWarningObjRelActiveCondCalc/1(ObjectMovingState)  "<ObjectMovingState>"
  Bus Selector/15 -> vehPreWarningObjRelActiveCondCalc/15(dxVariance)  "<dxVariance>"
  Bus Selector/10 -> vehPreWarningObjRelActiveCondCalc/10(vehConfigAEBPed)  "<VehicleConfigAEBPed>"
  Bus Selector/12 -> vehPreWarningObjRelActiveCondCalc/12(ObjectID)  "<ObjectID>"
  Bus Selector/3 -> vehPreWarningObjRelActiveCondCalc/3(ObjectTypeCameraConfirmed)  "<ObjectTypeCameraConfirmed>"
  Bus Selector/11 -> vehPreWarningObjRelActiveCondCalc/11(ObjectMovingOrientation)  "<ObjectMovingOrientation>"
  vehPreWarningSituationRelActiveCondCalc/1(vehPreWarningSituationRelActiveCondFulfilled) -> vehPreWarningSuppCondFinalJudge/3(vehPreWarningSituationRelActiveCondFulfilled)  "AEB_vehPreWarningSitRelActiveCond"
  vehPreWarningObjRelActiveCondCalc/1(vehPreWarningObjRelActiveCondFulfilled) -> vehPreWarningSuppCondFinalJudge/1(vehPreWarningObjRelActiveCondFulfilled)  "AEB_vehPreWarningObjActiveCond"
  vehPreWarningVehRelActiveCondCalc/1(vehPreWarningVehRelActiveCondFulfilled) -> vehPreWarningSuppCondFinalJudge/2(vehPreWarningVehRelActiveCondFulfilled)  "AEB_vehPreWarningVehRelActiveCond"
  vehPreWarningSuppCondFinalJudge/1(vehPreWarningSuppCond) -> vehPreWarningSuppCond/1
  Bus Selector1/3 -> vehPreWarningVehRelActiveCondCalc/3(vehStateCalcPreWarningSuppFlg)  "<VSCvehPreWarningSuppCond>"
  Bus Selector1/10 -> vehPreWarningVehRelActiveCondCalc/10(AccrPedlPosnDiagc)  "<AccrPedlPosnDiagc>"
  Bus Selector1/4 -> vehPreWarningVehRelActiveCondCalc/4(SteerWheelAngle)  "<SteerWheelAngle>"
  Bus Selector1/2 -> vehPreWarningVehRelActiveCondCalc/2(ObjectMovingState)  "<ObjectMovingState>"
  Bus Selector1/7 -> vehPreWarningVehRelActiveCondCalc/7(ESPmasterCylindBrakePress)  "<ESP_MasterCylindBrakePress>"
  Bus Selector1/1 -> vehPreWarningVehRelActiveCondCalc/1(subjVel)  "<subjVel>"
  Bus Selector1/8 -> vehPreWarningVehRelActiveCondCalc/8(BrakePedalStatus_G)  "<BrakePedalStatus_G>"
  Bus Selector1/5 -> vehPreWarningVehRelActiveCondCalc/5(SteerWheelSpd)  "<SteerWheelSpd>"
  inputSignals/1 -> Bus Selector/1, Bus Selector1/1, Bus Selector2/1
  Bus Selector2/7 -> vehPreWarningSituationRelActiveCondCalc/7(FcwHMISetting)  "<FCWSnvty>"
  Bus Selector2/9 -> vehPreWarningSituationRelActiveCondCalc/9(ACCModSts)  "<ACCModSts>"

== system TosAeb/FcwAebJa_Step_sys/ALG_AEBSystem/ALG_AEBController/AEB_FuncCondCalcApp/LongFunctionCondCalc/vehPrefillSuppAborCondCalc/vehPrefillAborCondCalc ==
  inputSignals : Inport
  Bus Selector : BusSelector  OutputSignals=longObjCalcInfo.LongObjRawInfo.ObjectID
  Bus Selector1 : BusSelector  OutputSignals=vehCanInfo.SteerWheelAngle,vehCanInfo.SteerWheelSpd,vehCanInfo.ESP_MasterCylindBrakePress,vehCanInfo.BrakePedalStatus_G,vehCanInfo.AccrPedlPosnDiagc,vehStateCalcInfo.VSCVehSuppAborCond.VSCvehPrefillAborCond
  Bus Selector2 : BusSelector  OutputSignals=longObjCalcInfo.LongCollisionAssess.longCollisionSeverity
  vehPrefillAborCondFinalJudge : Subsystem
  vehPrefillObjRelAborCondCalc : Subsystem
  vehPrefillSituationRelAborCondCalc : Subsystem
  vehPrefillVehRelAborCondCalc : Subsystem
  vehPrefillAborCondFulfilled : Outport
  wires:
  Bus Selector2/1 -> vehPrefillSituationRelAborCondCalc/1(longCollisionSeverity)  "<longCollisionSeverity>"
  Bus Selector1/6 -> vehPrefillVehRelAborCondCalc/6(vehStateCalcAcuteWarningAborFlg)  "<VSCvehPrefillAborCond>"
  Bus Selector1/5 -> vehPrefillVehRelAborCondCalc/5(AccrPedlPosnDiagc)  "<AccrPedlPosnDiagc>"
  Bus Selector1/4 -> vehPrefillVehRelAborCondCalc/4(BrakePedalStatus_G)  "<BrakePedalStatus_G>"
  Bus Selector1/1 -> vehPrefillVehRelAborCondCalc/1(SteerWheelAngle)  "<SteerWheelAngle>"
  Bus Selector1/3 -> vehPrefillVehRelAborCondCalc/3(ESPmasterCylindBrakePress)  "<ESP_MasterCylindBrakePress>"
  Bus Selector1/2 -> vehPrefillVehRelAborCondCalc/2(SteerWheelSpd)  "<SteerWheelSpd>"
  Bus Selector/1 -> vehPrefillObjRelAborCondCalc/1(ObjectID)  "<ObjectID>"
  inputSignals/1 -> Bus Selector/1, Bus Selector1/1, Bus Selector2/1
  vehPrefillAborCondFinalJudge/1(vehPrefillAborCondFulfilled) -> vehPrefillAborCondFulfilled/1
  vehPrefillSituationRelAborCondCalc/1(vehPrefillSituationRelAborCondFulfilled) -> vehPrefillAborCondFinalJudge/3(vehPrefillSituationRelAborCondFulfilled)  "AEB_vehPrefillSitRelAborCond"
  vehPrefillVehRelAborCondCalc/1(vehPrefillVehRelAborCondFulfilled) -> vehPrefillAborCondFinalJudge/2(vehPrefillVehRelAborCondFulfilled)  "AEB_vehPrefillVehRelAborCond"
  vehPrefillObjRelAborCondCalc/1(vehPrefillObjRelAborCondFulfilled) -> vehPrefillAborCondFinalJudge/1(vehPrefillObjRelAborCondFulfilled)  "AEB_vehPrefillObjRelAborCond"

== system TosAeb/FcwAebJa_Step_sys/ALG_AEBSystem/ALG_AEBController/AEB_FuncCondCalcApp/LongFunctionCondCalc/vehPrefillSuppAborCondCalc/vehPrefillSuppCondCalc ==
  inputSignals : Inport
  Bus Selector : BusSelector  OutputSignals=longObjCalcInfo.LongObjRawInfo.ObjectMovingState,longObjCalcInfo.LongObjRawInfo.ObjectTypeCameraConfirmed,vehStateCalcInfo.VSC_AEBFuncSwtSts.AEB_PedResp,longObjCalcInfo.LongObjRawInfo.ObjectType,subjInfo.subjCurvTraj,subjInfo.subjLatAccel,subjInfo.subjVel,vehConfigInfo.VehicleConfigAEBCyc,vehConfigInfo.VehicleConfigAEBPed,longObjCalcInfo.LongObjRawInfo.ObjectMovingOrientation,longObjCalcInfo.LongObjRawInfo.ObjectID,longObjCalcInfo.LongObjRawInfo.ObjectExistProb,longObjCalcInfo.LongObjRawInfo.ObjectIsObstacleProb,longObjCalcInfo.longObjAbsInfo.ObjFusQuality
  Bus Selector1 : BusSelector  OutputSignals=subjInfo.subjVel,longObjCalcInfo.LongObjRawInfo.ObjectMovingState,vehCanInfo.SteerWheelAngle,vehCanInfo.SteerWheelSpd,vehConfigInfo.VehConfigIbooster,vehCanInfo.ESP_MasterCylindBrakePress,vehCanInfo.BrakePedalStatus_G,vehCanInfo.IB_BrkPedalAppld,vehCanInfo.AccrPedlPosnDiagc,vehStateCalcInfo.VSCVehSuppAborCond.VSCvehPrefillSuppCond
  Bus Selector2 : BusSelector  OutputSignals=longObjCalcInfo.LongCollisionAssess.longCollisionSeverity,longObjCalcInfo.overlapRatio,longObjCalcInfo.longObjAbsInfo.dxFrontBumper,longObjCalcInfo.probEscape
  vehPrefillObjRelActiveCondCalc : Subsystem
  vehPrefillSituationRelActiveCondCalc : Subsystem
  vehPrefillSuppCondFinalJudge : Subsystem
  vehPrefillVehRelActiveCondCalc : Subsystem
  vehPrefillSuppCondFulfilled : Outport
  wires:
  Bus Selector1/5 -> vehPrefillVehRelActiveCondCalc/5(VehConfigIbooster)  "<VehConfigIbooster>"
  Bus Selector/14 -> vehPrefillObjRelActiveCondCalc/14(ObjFusQuality)  "<ObjFusQuality>"
  Bus Selector1/8 -> vehPrefillVehRelActiveCondCalc/8(IB_BrkPedalAppld)  "<IB_BrkPedalAppld>"
  Bus Selector2/4 -> vehPrefillSituationRelActiveCondCalc/4(probEscape)  "<probEscape>"
  Bus Selector2/1 -> vehPrefillSituationRelActiveCondCalc/1(longCollisionSeverity)  "<longCollisionSeverity>"
  Bus Selector2/3 -> vehPrefillSituationRelActiveCondCalc/3(dxFrontBumper)  "<dxFrontBumper>"
  Bus Selector2/2 -> vehPrefillSituationRelActiveCondCalc/2(overlapRatio)  "<overlapRatio>"
  Bus Selector1/1 -> vehPrefillVehRelActiveCondCalc/1(subjVel)  "<subjVel>"
  Bus Selector1/10 -> vehPrefillVehRelActiveCondCalc/10(vehStateCalcPrefillSuppFlg)  "<VSCvehPrefillSuppCond>"
  Bus Selector1/2 -> vehPrefillVehRelActiveCondCalc/2(ObjectMovingState)  "<ObjectMovingState>"
  Bus Selector1/9 -> vehPrefillVehRelActiveCondCalc/9(AccrPedlPosnDiagc)  "<AccrPedlPosnDiagc>"
  Bus Selector1/3 -> vehPrefillVehRelActiveCondCalc/3(SteerWheelAngle)  "<SteerWheelAngle>"
  Bus Selector1/7 -> vehPrefillVehRelActiveCondCalc/7(BrakePedalStatus_G)  "<BrakePedalStatus_G>"
  Bus Selector1/4 -> vehPrefillVehRelActiveCondCalc/4(SteerWheelSpd)  "<SteerWheelSpd>"
  Bus Selector1/6 -> vehPrefillVehRelActiveCondCalc/6(ESPmasterCylindBrakePress)  "<ESP_MasterCylindBrakePress>"
  Bus Selector/13 -> vehPrefillObjRelActiveCondCalc/13(ObjectIsObstacleProb)  "<ObjectIsObstacleProb>"
  Bus Selector/12 -> vehPrefillObjRelActiveCondCalc/12(ObjectExistProb)  "<ObjectExistProb>"
  Bus Selector/11 -> vehPrefillObjRelActiveCondCalc/11(ObjectID)  "<ObjectID>"
  Bus Selector/1 -> vehPrefillObjRelActiveCondCalc/1(ObjectMovingState)  "<ObjectMovingState>"
  Bus Selector/10 -> vehPrefillObjRelActiveCondCalc/10(ObjectMovingOrientation)  "<ObjectMovingOrientation>"
  Bus Selector/2 -> vehPrefillObjRelActiveCondCalc/2(ObjectTypeCameraConfirmed)  "<ObjectTypeCameraConfirmed>"
  Bus Selector/9 -> vehPrefillObjRelActiveCondCalc/9(vehConfigAEBPed)  "<VehicleConfigAEBPed>"
  Bus Selector/3 -> vehPrefillObjRelActiveCondCalc/3(AEB_PedResp)  "<AEB_PedResp>"
  Bus Selector/8 -> vehPrefillObjRelActiveCondCalc/8(vehConfigAEBCyc)  "<VehicleConfigAEBCyc>"
  Bus Selector/4 -> vehPrefillObjRelActiveCondCalc/4(ObjectType)  "<ObjectType>"
  Bus Selector/7 -> vehPrefillObjRelActiveCondCalc/7(subjVel)  "<subjVel>"
  Bus Selector/5 -> vehPrefillObjRelActiveCondCalc/5(subjCurvTraj)  "<subjCurvTraj>"
  Bus Selector/6 -> vehPrefillObjRelActiveCondCalc/6(subjLatAccel)  "<subjLatAccel>"
  inputSignals/1 -> Bus Selector/1, Bus Selector1/1, Bus Selector2/1
  vehPrefillSituationRelActiveCondCalc/1(vehPrefillSituationRelActiveCondFulfilled) -> vehPrefillSuppCondFinalJudge/3(vehPrefillSituationRelActiveCondFulfilled)  "AEB_vehPrefillSituationRelActiveCond"
  vehPrefillObjRelActiveCondCalc/1(vehPrefillObjRelActiveCondFulfilled) -> vehPrefillSuppCondFinalJudge/1(vehPrefillObjRelActiveCondFulfilled)  "AEB_vehPrefillObjRelActiveCond"
  vehPrefillVehRelActiveCondCalc/1(vehPrefillVehRelActiveCondFulfilled) -> vehPrefillSuppCondFinalJudge/2(vehPrefillVehRelActiveCondFulfilled)  "AEB_vehPrefillVehRelActiveCond"
  vehPrefillSuppCondFinalJudge/1(vehPrefillSuppCondFulfilled) -> vehPrefillSuppCondFulfilled/1  "vehPrefillSuppCondFulfilled"

== system TosAeb/FcwAebJa_Step_sys/ALG_AEBSystem/ALG_AEBController/AEB_FuncCondCalcApp/PedFunctionCondCalc/pedABASuppAborCond/pedABAAborCondCalc ==
  inputSignals : Inport
  Bus Selector : BusSelector  OutputSignals=latPedTgtCalcInfo.latPedObjCalcInfo.latPedObjRawInfo.ObjectID
  Bus Selector1 : BusSelector  OutputSignals=vehStateCalcInfo.VSCPedFuncSuppAborCond.VSCPedABAAborCond,latPedTgtCalcInfo.latPedCollsionAssesInfo.latCollisionSeverity,vehCanInfo.SteerWheelAngle,vehCanInfo.SteerWheelSpd
  Bus Selector2 : BusSelector  OutputSignals=latPedTgtCalcInfo.latPedCollsionAssesInfo.latCollisionSeverity,latFuncActiveStateTime.pedABAWorkTime
  pedABAAborCondFinalJudge : Subsystem
  pedABAObjRelActiveCondCalc : Subsystem
  pedABASituationRelAborCondCalc : Subsystem
  pedABAVehRelAborCondCalc : Subsystem
  pedABAAborCondFulfilled : Outport
  wires:
  Bus Selector1/4 -> pedABAVehRelAborCondCalc/4(SteerWheelSpd)  "<SteerWheelSpd>"
  Bus Selector1/3 -> pedABAVehRelAborCondCalc/3(SteerWheelAngle)  "<SteerWheelAngle>"
  Bus Selector1/2 -> pedABAVehRelAborCondCalc/2(latCollisionSeverity)  "<latCollisionSeverity>"
  Bus Selector1/1 -> pedABAVehRelAborCondCalc/1(vehStateCalcABAAborFlg)  "<VSCPedABAAborCond>"
  pedABAAborCondFinalJudge/1(pedABAAborCondFulfilled) -> pedABAAborCondFulfilled/1
  pedABASituationRelAborCondCalc/1(pedABASituationRelAborCondFulfilled) -> pedABAAborCondFinalJudge/3(pedABASituationRelAborCondFulfilled)  "AEB_pedABASitRelAborCond"
  pedABAVehRelAborCondCalc/1(pedABAVehRelAborCondFulfilled) -> pedABAAborCondFinalJudge/2(pedABAVehRelAborCondFulfilled)  "AEB_pedABAVehRelAborCond"
  pedABAObjRelActiveCondCalc/1(pedABAObjRelAborCondFulfilled) -> pedABAAborCondFinalJudge/1(pedABAObjRelAborCondFulfilled)  "AEB_pedABAObjRelAborCond"
  Bus Selector/1 -> pedABAObjRelActiveCondCalc/1(ObjectID)  "<ObjectID>"
  inputSignals/1 -> Bus Selector/1, Bus Selector2/1, Bus Selector1/1
  Bus Selector2/1 -> pedABASituationRelAborCondCalc/1(latCollisionSeverity)  "<latCollisionSeverity>"
  Bus Selector2/2 -> pedABASituationRelAborCondCalc/2(pedABAWorkTime)  "<pedABAWorkTime>"

== system TosAeb/FcwAebJa_Step_sys/ALG_AEBSystem/ALG_AEBController/AEB_FuncCondCalcApp/PedFunctionCondCalc/pedABASuppAborCond/pedABASuppCondCalc ==
  inputSignals : Inport
  Bus Selector : BusSelector  OutputSignals=latPedTgtCalcInfo.latPedObjCalcInfo.latPedObjRawInfo.ObjectID,latPedTgtCalcInfo.latPedObjCalcInfo.latPedObjAbsInfo.vxAbsObj,latPedTgtCalcInfo.latPedObjCalcInfo.latPedObjAbsInfo.ObjFusQuality,latPedTgtCalcInfo.latPedObjCalcInfo.latPedObjRawInfo.dy,latPedTgtCalcInfo.latPedObjCalcInfo.latPedObjRawInfo.vy
  Bus Selector1 : BusSelector  OutputSignals=subjInfo.subjVel,vehCanInfo.SteerWheelAngle,vehCanInfo.SteerWheelSpd,vehCanInfo.AccrPedlPosnDiagc,vehStateCalcInfo.VSCPedFuncSuppAborCond.VSCPedABASuppCond
  Bus Selector2 : BusSelector  OutputSignals=latPedTgtCalcInfo.latPedCollsionAssesInfo.latCollisionSeverity,subjInfo.subjCurvTraj,subjInfo.subjLatAccel,latPedTgtCalcInfo.latPedObjCalcInfo.latPedObjRawInfo.ObjectTypeCameraConfirmed,latPedTgtCalcInfo.latPedObjCalcInfo.latPedObjRawInfo.vy,latPedTgtCalcInfo.latPedObjCalcInfo.latPedObjAbsInfo.dxFrontBumper,latPedTgtCalcInfo.latPedObjCalcInfo.CollisionRiskProb,vehStateCalcInfo.VSC_AEBFuncSwtSts.FCWSnvty,subjInfo.subjVel
  pedABAObjRelActiveCondCalc : Subsystem
  pedABASituationRelActiveCondCalc : Subsystem
  pedABASuppCondFinalJudge : Subsystem
  pedABAVehRelActiveCondCalc : Subsystem
  pedABASuppCondFulfilled : Outport
  wires:
  Bus Selector/5 -> pedABAObjRelActiveCondCalc/5(vy)  "<vy>"
  Bus Selector/4 -> pedABAObjRelActiveCondCalc/4(dy)  "<dy>"
  Bus Selector/3 -> pedABAObjRelActiveCondCalc/3(ObjFusQuality)  "<ObjFusQuality>"
  Bus Selector/2 -> pedABAObjRelActiveCondCalc/2(vxAbsObj)  "<vxAbsObj>"
  Bus Selector2/1 -> pedABASituationRelActiveCondCalc/1(latCollisionSeverity)  "<latCollisionSeverity>"
  Bus Selector2/9 -> pedABASituationRelActiveCondCalc/9(subjVel)  "<subjVel>"
  Bus Selector2/8 -> pedABASituationRelActiveCondCalc/8(FcwHMISetting)  "<FCWSnvty>"
  Bus Selector2/6 -> pedABASituationRelActiveCondCalc/6(dxFrontBumper)  "<dxFrontBumper>"
  Bus Selector2/5 -> pedABASituationRelActiveCondCalc/5(vy)  "<vy>"
  Bus Selector2/4 -> pedABASituationRelActiveCondCalc/4(ObjectTypeCameraConfirmed)  "<ObjectTypeCameraConfirmed>"
  Bus Selector2/3 -> pedABASituationRelActiveCondCalc/3(subjLatAccel)  "<subjLatAccel>"
  Bus Selector2/2 -> pedABASituationRelActiveCondCalc/2(subjCurvTraj)  "<subjCurvTraj>"
  Bus Selector2/7 -> pedABASituationRelActiveCondCalc/7(colliisonRiskProb)  "<CollisionRiskProb>"
  Bus Selector1/5 -> pedABAVehRelActiveCondCalc/5(vehStateCalcPedABASuppFlg)  "<VSCPedABASuppCond>"
  Bus Selector1/4 -> pedABAVehRelActiveCondCalc/4(AccrPedlPosnDiagc)  "<AccrPedlPosnDiagc>"
  Bus Selector1/3 -> pedABAVehRelActiveCondCalc/3(SteerWheelSpd)  "<SteerWheelSpd>"
  Bus Selector1/2 -> pedABAVehRelActiveCondCalc/2(SteerWheelAngle)  "<SteerWheelAngle>"
  Bus Selector1/1 -> pedABAVehRelActiveCondCalc/1(subjVel)  "<subjVel>"
  pedABASituationRelActiveCondCalc/1(pedABASituationRelSuppCondFulfilled) -> pedABASuppCondFinalJudge/3(pedABASituationRelSuppCondFulfilled)  "AEB_pedABASituationRelSuppCond"
  pedABAObjRelActiveCondCalc/1(pedABAObjRelSuppCondFulfilled) -> pedABASuppCondFinalJudge/1(pedABAObjRelSuppCondFulfilled)  "AEB_pedABAObjRelSuppCond"
  pedABAVehRelActiveCondCalc/1(pedABAVehRelSuppCondFulfilled) -> pedABASuppCondFinalJudge/2(pedABAVehRelSuppCondFulfilled)  "AEB_pedABAVehRelSuppCond"
  pedABASuppCondFinalJudge/1(pedABASuppCondFulfilled) -> pedABASuppCondFulfilled/1
  Bus Selector/1 -> pedABAObjRelActiveCondCalc/1(ObjectID)  "<ObjectID>"
  inputSignals/1 -> Bus Selector/1, Bus Selector1/1, Bus Selector2/1
